simulink model "ospfb_2x_64kc_8i_16o"  (R2021a)
settings: Solver=VariableStepAuto  StartTime=0.0  StopTime=2304  RelTol=1e-3
undefined symbols (not defined in the file or in its folder): pfb_coeff_gen_calc
libraries: xbsIndex_r4 x37193, casper_library_misc x226, casper_library_delays x184
(part 201 of 313)

== system ospfb_2x_64kc_8i_16o/fft2/fft_direct/butterfly3_7/twiddle/coeff_gen/cosin ==
  theta : Inport
  misci : Inport  Port=2
  Constant4 : xbsIndex_r4/Constant  const=0  gui_display_data_type=Fixed-point  arith_type=Unsigned  n_bits=1  bin_pt=0  preci_type=Single  exp_width=8  frac_width=24  explicit_period=on  period=1  equ=P=C  opselect=C  inp2=PCIN>>17  opr=+  inp1=P  carry=CIN  dbl_ovrd=off  block_type=constant
  Constant5 : xbsIndex_r4/Constant  const=0  gui_display_data_type=Fixed-point  arith_type=Unsigned  n_bits=1  bin_pt=0  preci_type=Single  exp_width=8  frac_width=24  explicit_period=on  period=1  equ=P=C  opselect=C  inp2=PCIN>>17  opr=+  inp1=P  carry=CIN  dbl_ovrd=off  block_type=constant
  Delay : xbsIndex_r4/Delay  rst=off  infoeditControl=Selection of Reset will increase slice count due to use of real FFs and instead of SRLs  en=off  latency=bram_latency  reg_retiming=on  dbl_ovrd=off  block_type=delay
  Delay10 : xbsIndex_r4/Delay  rst=off  infoeditControl=Selection of Reset will increase slice count due to use of real FFs and instead of SRLs  en=off  latency=bram_latency  reg_retiming=on  dbl_ovrd=off  block_type=delay
  Delay8 : xbsIndex_r4/Delay  rst=off  infoeditControl=Selection of Reset will increase slice count due to use of real FFs and instead of SRLs  en=off  latency=bram_latency  reg_retiming=on  dbl_ovrd=off  block_type=delay
  ROM : xbsIndex_r4/ROM  depth=2^(12)  initVector=[36975361041 36974050333 36973001768 36971691060 36970380351 36969069643 36967758935 36966710370 36965399662 36964088953 36962778245 36961467537 36960418972 36959108264 36957797555 36956486847 36955176139 36954127574 36952816866 36951506158 36950195449 36949146885 36947836176 36946525468 36945214760 36943904051 36942855487 36941544779 36940234070 36938923362 36937612653 36936564089 36935253381 36933942672 36932631964 36931583400 36930272691 36928961983 36927651274 36926602710 36925292002 36923981293 36922670585 36921622021 36920311312 36919000604 36917689895 36916379187 36915330623 36914019914 36912709206 36911398498 36910349933 36909039225 36907728517 36906417808 36905369244 36904058535 36902747827 36901437119 36900388554 36899077846 36897767138 36896718573 36895407865 36894097157 36892786448 36891737884 36890427175 36889116467 36887805759 36886757194 36885446486 36884135778 36882825069 36881776505 36880465797 36879155088 36878106524 36876795816 36875485107 36874174399 36873125834 36871815126 36870504418 36869455853 36868145145 36866834437 36865523728 36864475164 36863164456 36861853747 36860805183 36859494475 36858183766 36856873058 36855824494 36854513785 36853203077 36852154513 36850843804 36849533096 36848484531 36847173823 36845863115 36844552406 36843503842 36842193134 36840882425 36839833861 36838523153 36837212444 36836163880 36834853172 36833542463 36832493899 36831183191 36829872482 36828823918 36827513210 36826202501 36825153937 36823843229 36822532520 36821483956 36820173248 36818862539 36817813975 36816503267 36815192558 36814143994 36812833286 36811522577 36810474013 36809163305 36807852596 36806804032 36805493324 36804182615 36803134051 36801823343 36800512634 36799464070 36798153362 36796842653 36795794089 36794483381 36793172672 36792124108 36790813400 36789502692 36788454127 36787143419 36786094855 36784784146 36783473438 36782424874 36781114165 36779803457 36778754893 36777444184 36776133476 36775084912 36773774203 36772725639 36771414931 36770104222 36769055658 36767744950 36766434241 36765385677 36764074969 36763026405 36761715696 36760404988 36759356424 36758045715 36756997151 36755686443 36754375734 36753327170 36752016462 36750967897 36749657189 36748346481 36747297917 36745987208 36744676500 36743627936 36742317227 36741268663 36739957955 36738909390 36737598682 36736287974 36735239409 36733928701 36732880137 36731569429 36730258720 36729210156 36727899448 36726850883 36725540175 36724229467 36723180902 36721870194 36720821630 36719510922 36718462357 36717151649 36715840941 36714792376 36713481668 36712433104 36711122396 36710073831 36708763123 36707452415 36706403850 36705093142 36704044578 36702733869 36701685305 36700374597 36699326033 36698015324 36696704616 36695656052 36694345343 36693296779 36691986071 36690937507 36689626798 36688578234 36687267526 36685956817 36684908253 36683597545 36682548981 36681238272 36680189708 36678879000 36677830435 36676519727 36675471163 36674160455 36673111890 36671801182 36670490474 36669441909 36668131201 36667082637 36665771929 36664723364 36663412656 36662364092 36661053384 36660004819 36658694111 36657645547 36656334838 36655286274 36653975566 36652927002 36651616293 36650567729 36649257021 36648208457 36646897748 36645849184 36644538476 36643489911 36642179203 36641130639 36639819931 36638771366 36637460658 36636412094 36635101386 36634052821 36632742113 36631693549 36630382841 36629334276 36628023568 36626975004 36625664295 36624615731 36623305023 36622256459 36620945750 36619897186 36618586478 36617537914 36616227205 36615178641 36613867933 36612819369 36611508660 36610460096 36609149388 36608100824 36606790115 36605741551 36604692987 36603382279 36602333714 36601023006 36599974442 36598663734 36597615169 36596304461 36595255897 36593945189 36592896624 36591585916 36590537352 36589488788 36588178079 36587129515 36585818807 36584770243 36583459534 36582410970 36581100262 36580051698 36579003133 36577692425 36576643861 36575333153 36574284588 36572973880 ...(+45156 chars)]  distributed_mem=Block RAM  rst=off  init_reg=0  en=off  latency=bram_latency  gui_display_data_type=Fixed-point  arith_type=Unsigned  n_bits=n_bits*2  bin_pt=0  preci_type=Single  exp_width=8  frac_width=24  optimize=Speed  use_rpm=on  dbl_ovrd=off  simulation_type=fast_sim  block_type=sprom
  Terminator : Terminator
  Terminator1 : Terminator
  Terminator4 : Terminator
  add_convert0 : Subsystem
  add_convert1 : Subsystem
  assert : xbsIndex_r4/Assert  assert_type=on  type_source=Explicitly  gui_display_data_type=Fixed-point  arith_type=Unsigned  n_bits=12  bin_pt=0  preci_type=Single  exp_width=8  frac_width=24  assert_rate=off  rate_source=Explicitly  period=1  output_port=on  block_type=assert
  c_to_ri : casper_library_misc/c_to_ri  n_bits=n_bits  bin_pt=bin_pt
      settings: SourceProductName=CASPER DSP Blockset
  invert0 : Subsystem  mask{n_bits=n_bits, bin_pt=bin_pt, neg_latency=neg_latency, mux_latency=mux_latency}
      mask prompts: n_bits "bit width"; bin_pt "binary point"; neg_latency "negate latency"; mux_latency "mux latency"
  invert1 : Subsystem  mask{n_bits=n_bits, bin_pt=bin_pt, neg_latency=neg_latency, mux_latency=mux_latency}
      mask prompts: n_bits "bit width"; bin_pt "binary point"; neg_latency "negate latency"; mux_latency "mux latency"
  -sin : Outport
  -cos : Outport  Port=2
  misco : Outport  Port=3
  wires:
  theta/1 -> assert/1
  assert/1 -> add_convert0/1(theta), add_convert1/1(theta)
  Constant4/1 -> add_convert0/2(misci)
  add_convert0/3(misco) -> Terminator/1
  misci/1 -> add_convert1/2(misci)
  add_convert1/3(misco) -> Delay/1
  add_convert0/2(add) -> ROM/1
  add_convert1/2(add) -> Terminator4/1
  ROM/1 -> c_to_ri/1
  add_convert1/1(negate) -> Delay8/1
  add_convert0/1(negate) -> Delay10/1
  Delay10/1 -> invert0/1(negate)
  Constant5/1 -> invert0/3(misci)
  c_to_ri/1 -> invert0/2(in)
  Delay8/1 -> invert1/1(negate)
  c_to_ri/2 -> invert1/2(in)
  invert0/2(misco) -> Terminator1/1
  Delay/1 -> invert1/3(misci)
  invert0/1(out) -> -sin/1
  invert1/1(out) -> -cos/1
  invert1/2(misco) -> misco/1
